simulink model "GLOSA_cs"  (Simulink 7.6)
settings: Solver=ode5  SolverMode=SingleTasking  StartTime=0.0  StopTime=40  FixedStep=1/100  RelTol=1e-3
PreLoadFcn:
  | check_NUTS_defined;
  | 
  | assignin('base',['model_closed_' bdroot],false);
InitFcn:
  | PreScanExperimentInitFcn(bdroot)
StopFcn:
  | PreScanExperimentStopFcn(bdroot)
undefined symbols (not defined in the file or in its folder): acceleration, actorID, actorType, DiscIntNeverNeededParam, GPS_alt, GPS_lat, GPS_long, intmax, lightBarInUse, messageID, publicVehicleType, rotZ, sireneInUse, trafficLightPriority, turnAdvice, velocity, x_ini_VRML, y_ini_VRML, yawRate, z_ini_VRML
libraries: simulink x337, simulink_extras x42, PreScanSimulinkLibrary x38, PreScanUsefulBlocks x14, simulink_need_slupdate x6
(part 38 of 38)

== stateflow chart Toyota_Previa_1/GLOSA/GLOSA ==
  state GLOSA [parallel]
    settings: executionOrder=1
    state dvVelocity
      (default) -> (junction 1368) : /* Velocity */
      (junction 1368) -> (junction 1370) [order 1] : [ (vForward == 1)]
      (junction 1370) -> (junction 1380) : {dvVelocity = CAM_Velocity[i];}
          | /* If car is at the front desired velocity if cars are set to its value */
      (junction 1368) -> (junction 1314) [order 2] : {dvVelocity = desiredVelocity;}
      (junction 1380) -> (junction 1314)
      (junction 1314) -> (junction 1381)
    state AngleandDistance
      state vDistance [parallel]
        settings: executionOrder=2
        (junction 1362) -> (junction 1361) : {Distance = 6371000*c;}
            | /*Calculate distance to the object */
        (default) -> (junction 1363) : {a = (sin((CAM_GPSLatitude[i]*d2r-selfGPSLatitude*d2r)/2 ))^2+ cos(selfGPSLatitude*d2r)*cos(CAM_GPSLatitude[i]*d2r)*(sin((CAM_GPSLongitude[i]*d2r-selfGPSLongitude*d2r)/2))^2;}
        (junction 1363) -> (junction 1362) : {c=2*atan2(sqrt(a), sqrt(1-a));}
      state vAngle [parallel]
        settings: executionOrder=1
        (default) -> (junction 1364) : {Angle = (atan2(CAM_GPSLatitude[i]-selfGPSLatitude, CAM_GPSLongitude[i] - selfGPSLongitude))/d2r;}
            | /* Calculate angle to the object */
    state diVelocity
      (default) -> (junction 1335) : /* Velocity */
      (junction 1316) -> (junction 1369)
      (junction 1335) -> (junction 1334) [order 1] : [ (iForward == 1)]
      (junction 1335) -> (junction 1316) [order 2] : {diVelocity = desiredVelocity;}
      (junction 1334) -> (junction 1367) : {diVelocity = (CAM_message[i] - 1000) /3.6;}
          | /* If sign is foreward, set desired infrastructure velocity to its limit */
      (junction 1367) -> (junction 1316)
    state vForward
      (junction 1320) -> (junction 1326) [order 1] : [((CAM_YawRate[i] > 2 || CAM_YawRate[i] <-2)  && (selfYawRate > 2 || selfYawRate < -2))]
      (junction 1326) -> (junction 1350) : {R1 = CAM_Velocity[i]/(CAM_YawRate[i]*d2r);
          | R2 = selfVelocity/(selfYawRate*d2r);
          | alfa = CAM_Heading[i]-selfHeading;
          | L = 2*d2r*180*R2*(alfa/360);}
      (junction 1387) -> (junction 1372)
      (junction 1371) -> (junction 1387) : {bend = 1;}
          | /* Cars are on the same bent if both have non-zero yaw rate and estimated agle at detected distance of the host car is similar to the angle of detected vehicle */
      (junction 1350) -> (junction 1372) [order 2] : {bend = 2;}
      (junction 1310) -> (junction 1313)
      (junction 1324) -> (junction 1311) [order 2]
      (junction 1372) -> (junction 1325)
      (junction 1325) -> (junction 1324)
      (junction 1313) -> (junction 1311) [order 2]
      (default) -> (junction 1320)
      (junction 1317) -> (junction 1318) [order 1] : [abs((Angle-selfHeading)%%360)<45 || abs((Angle-selfHeading)%%360)>315 || bend == 1]
      (junction 1366) -> (junction 1358) : {vForward = 0;}
      (junction 1318) -> (junction 1319) : {vForward = 1;}
          | /* Car is at the front if it will be reached in 2s and angles of cars are similar or they are on the bend */
      (junction 1324) -> (junction 1310) [order 1] : [(abs(CAM_Heading[i]-selfHeading)<45 || abs(CAM_Heading[i]-selfHeading)>315) || bend == 1 ]
      (junction 1317) -> (junction 1366) [order 2]
      (junction 1313) -> (junction 1315) [order 1] : [Distance < 100 && (selfVelocity - CAM_Velocity[i]) > 0 &&((Distance / (selfVelocity - CAM_Velocity[i])) < 2) && (abs(selfGPSAltitude - CAM_GPSAltitude[i]) < 10)]
      (junction 1320) -> (junction 1325) [order 2] : {bend = 0;}
      (junction 1315) -> (junction 1317)
      (junction 1311) -> (junction 1366)
      (junction 1350) -> (junction 1371) [order 1] : [((abs(L/selfVelocity*selfYawRate + selfHeading - CAM_Heading[i]) < 45) || (abs(L/selfVelocity*selfYawRate + selfHeading - CAM_Heading[i]) > 315)) ]
    state Velocity
      (junction 1340) -> (junction 1338) [order 2]
      (junction 1340) -> (junction 1339) [order 1] : [(dvVelocity<diVelocity) && (dvVelocity < desiredVelocity) && (vForward == 1)]
      (junction 1342) -> (junction 1340)
      (junction 1341) -> (junction 1342)
      (junction 1356) -> (junction 1341) : {Velocity = diVelocity;}
          | /* When Velocity from the sign is smaller then actual velocity of the car, Desired host velocity is set to the sign limit */
      (junction 1357) -> (junction 1342) [order 2] : {Velocity = desiredVelocity;}
      (junction 1357) -> (junction 1356) [order 1] : [(diVelocity<desiredVelocity)]
      (default) -> (junction 1357) : /* Velocity */
      (junction 1339) -> (junction 1337) : {Velocity = dvVelocity;}
          | /* When detected car hes smaller velocity then host car and sign limits, desired velocity of host car is set to velocity of detected car */
      (junction 1338) -> (junction 1336) : {Velocity = min(dvVelocity, diVelocity, dlVelocity, desiredVelocity);}
      (junction 1337) -> (junction 1338)
    state lForward
      note: IF condition1 THEN action1
          | ELSE action2
          | ENDIF
      (junction 1377) -> (junction 1322) : {lForward = 0;}
      (junction 1393) -> (junction 1323) : {lForward = 1;}
          | /* Light recognized as foreward one, when it is less then 500m away and Angle to it less then 45 deg.*/
      (junction 1375) -> (junction 1393) [order 1] : [abs((Angle-selfHeading) %% 360)<45 || abs((Angle-selfHeading)%% 360)>315]
      (junction 1375) -> (junction 1377) [order 2]
      (junction 1390) -> (junction 1373) [order 2]
      (junction 1374) -> (junction 1373) [order 2]
      (junction 1379) -> (junction 1375)
      (junction 1389) -> (junction 1374)
      (junction 1374) -> (junction 1379) [order 1] : [Distance < 500 && (abs(selfGPSAltitude - CAM_GPSAltitude[i]) < 10)]
      (junction 1373) -> (junction 1377)
      (junction 1390) -> (junction 1389) [order 1] : [abs(CAM_Heading[i]-selfHeading)<45 || abs(CAM_Heading[i]-selfHeading)>315]
      (default) -> (junction 1390)
    state iForward
      note: IF condition1 THEN action1
          | ELSE action2
          | ENDIF
      (junction 1312) -> (junction 1359) [order 1] : [Distance < 20 && (abs(selfGPSAltitude - CAM_GPSAltitude[i]) < 10)]
      (junction 1321) -> (junction 1347) [order 1] : [abs((Angle-selfHeading)%%360)<45 || abs((Angle-selfHeading)%%360)>315]
      (junction 1312) -> (junction 1346) [order 2]
      (junction 1347) -> (junction 1348) : {iForward = 1;}
          | /* Sign recognized as foreward one, when it is less then 20m away and Angle to it less then 45 deg.*/
      (junction 1346) -> (junction 1360)
      (junction 1359) -> (junction 1321)
      (junction 1360) -> (junction 1349) : {iForward = 0;}
      (junction 1321) -> (junction 1360) [order 2]
      (junction 1365) -> (junction 1355) [order 1] : [abs(CAM_Heading[i]-selfHeading)<45 || abs(CAM_Heading[i]-selfHeading)>315]
      (default) -> (junction 1365)
      (junction 1355) -> (junction 1312)
      (junction 1365) -> (junction 1346) [order 2]
    state dlVelocity
      (junction 1353) -> (junction 1351) : {dlVelocity = max((Distance-7)/(CAM_warning[i]+20), 0);}
          | /*dlVelocity = Distance/CAM_warning[i];}*/
      (junction 1384) -> (junction 1354) [order 2]
      (junction 1388) -> (junction 1391)
      (junction 1392) -> (junction 1388)
      (junction 1382) -> (junction 1388) [order 2] : {dlVelocity=desiredVelocity;}
      (junction 1378) -> (junction 1376)
      (junction 1386) -> (junction 1384) [order 2]
      (junction 1351) -> (junction 1392)
      (junction 1354) -> (junction 1353) [order 2]
      (junction 1354) -> (junction 1352) [order 1] : [TTL<CAM_warning[i]]
      (junction 1386) -> (junction 1385) [order 1] : [CAM_trafficLightPriority[i] == 3]
      (junction 1352) -> (junction 1376) : {dlVelocity= desiredVelocity;}
      (junction 1328) -> (junction 1378)
      (junction 1376) -> (junction 1351)
      (junction 1385) -> (junction 1328) : {dlVelocity=desiredVelocity;}
      (junction 1327) -> (junction 1378) : {dlVelocity = max((Distance-2)/(CAM_warning[i]+0.5), 0);}
      (junction 1384) -> (junction 1327) [order 1] : [CAM_trafficLightPriority[i] == 1]
      (default) -> (junction 1382) : /* Velocity */
      (junction 1382) -> (junction 1383) [order 1] : [ lForward == 1]
      (junction 1383) -> (junction 1386) : {TTL = Distance/selfVelocity;
          | Light = CAM_trafficLightPriority[i];
          | Time = CAM_warning[i];}
    lForward -> dlVelocity
    (junction 1330) -> AngleandDistance
    (default) -> (junction 1329) : {i =0;
        | vForward = 0;
        | iForward = 0;}
    (junction 1329) -> (junction 1330) [order 1] : [(vForward ==0 || iForward == 0) && i < CAM_Size]
        |  /*till sign and vehicle aren't detected*/
    (junction 1331) -> iForward [order 2] : [iForward == 0 && (CAM_vehicleType[i] == 14 || CAM_vehicleType[i] == 15) && CAM_message[i] > 1000]
        | /* If no traffic sign are detected and Velocity of the sign isn't 0 */
    (junction 1333) -> (junction 1329) : {i = 0;
        | vForward = 0;
        | iForward = 0;
        | lForward = 0;}
    (junction 1343) -> (junction 1329) : {i++;}
    (junction 1331) -> (junction 1332) [order 3]
    (junction 1329) -> Velocity [order 2]
    (junction 1332) -> vForward [order 1] : [vForward == 0 && CAM_vehicleType[i] != 0]
        | /* If there was no nearer car at the front and Vehicle Type ID isn't 0 */
    (junction 1332) -> (junction 1343) [order 2]
    iForward -> diVelocity
    Velocity -> (junction 1333)
    vForward -> dvVelocity
    dvVelocity -> (junction 1345)
    diVelocity -> (junction 1345)
    (junction 1344) -> (junction 1343)
    (junction 1345) -> (junction 1344)
    AngleandDistance -> (junction 1331)
    (junction 1331) -> lForward [order 1] : [lForward == 0 && CAM_trafficLightPriority[i] !=0]
    dlVelocity -> (junction 1345)
  note: GLOSA Algorithms Based on CAM signal changed to continous
  data: R1 local double; R2 local double; a local double; alfa local double; bend local double; c local double; diVelocity local double =100; dvVelocity local double =100; i local double; iForward local double; L local double; vForward local double; dlVelocity local double =100; selfHeading input double; selfGPSLongitude input double; selfGPSLatitude input double; selfGPSAltitude input double; selfYawRate input double; selfVelocity input double; CAM_warning input double; CAM_message input double; CAM_vehicleType input double; Velocity output double; CAM_Velocity input double; CAM_YawRate input double; CAM_trafficLightPriority input double; CAM_GPSLatitude input double; CAM_GPSLongitude input double; CAM_GPSAltitude input double; CAM_Heading input double; CAM_Size input double; d2r constant double =pi()/180; desiredVelocity input double; TTL local double; lForward output double; Angle local double; Distance local double; Time output double; Light output double

== matlab function Toyota_Previa_1/GLOSA/vehicle CAM generator/For Iterator Subsystem/Continuous signal ==
  data: CAM input double; CAM_o output double; CAM_old input double; i input double; NoMM input double; tmp_in input double; tmp_out output double
function [CAM_o, tmp_out] = con(CAM, CAM_old, i, NoMM, tmp_in)
%linearization and number of missed frames control
CAM_o = zeros(1, 16);
tmp_out = tmp_in(i);
if (CAM(i, 3) ~= 0)
    if (mod(tmp_in(i), NoMM+1) == 0)
        CAM_o = CAM (i, :);
    end
    tmp_out = tmp_in(i)+1;
else
    CAM_o = CAM_old(i, :);
end

== matlab function Toyota_Previa_1/GLOSA/vehicle CAM generator/Seperate signals ==
  data: CAM_warning output double; CAM input double; CAM_messageID output double; CAM_vehicleID output double; CAM_vehicleType output double; CAM_publicVehicleType output double; CAM_lightBarInUse output double; CAM_sireneInUse output double; CAM_velocity output double; CAM_acceleration output double; CAM_yawRate output double; CAM_turnAdvice output double; CAM_trafficLightPriority output double; CAM_GPSlat output double; CAM_GPSlong output double; CAM_GPSalt output double; CAM_rotZ output double
function [CAM_warning, CAM_messageID, CAM_vehicleID, CAM_vehicleType, CAM_publicVehicleType, CAM_lightBarInUse, CAM_sireneInUse, CAM_velocity, CAM_acceleration, CAM_yawRate, CAM_turnAdvice, CAM_trafficLightPriority, CAM_GPSlat, CAM_GPSlong, CAM_GPSalt, CAM_rotZ] = fcn(CAM)
%Seperate signals

CAM_warning = CAM(:, 1);
CAM_messageID = CAM(:, 2); 
CAM_vehicleID = CAM(:, 3);
CAM_vehicleType = CAM(:, 4);
CAM_publicVehicleType = CAM(:, 5);
CAM_lightBarInUse = CAM(:, 6);
CAM_sireneInUse = CAM(:, 7);
CAM_velocity = CAM(:, 8);
CAM_acceleration = CAM(:, 9);
CAM_yawRate = CAM(:, 10);
CAM_turnAdvice = CAM(:, 11);
CAM_trafficLightPriority = CAM(:, 12);
CAM_GPSlat = CAM(:, 13);
CAM_GPSlong = CAM(:, 14);
CAM_GPSalt = CAM(:, 15);
CAM_rotZ = CAM(:, 16);

== matlab function Toyota_Previa_1/GLOSA/vehicle CAM generator/Signal Matrix create ==
  data: CAM_warning input double; CAM output double; CAM_messageID input double; CAM_vehicleID input double; CAM_vehicleType input double; CAM_publicVehicleType input double; CAM_lightBarInUse input double; CAM_sireneInUse input double; CAM_velocity input double; CAM_acceleration input double; CAM_yawRate input double; CAM_turnAdvice input double; CAM_trafficLightPriority input double; CAM_GPSlat input double; CAM_GPSlong input double; CAM_GPSalt input double; CAM_rotZ input double
function CAM = fcn(CAM_warning, CAM_messageID, CAM_vehicleID, CAM_vehicleType, CAM_publicVehicleType, CAM_lightBarInUse, CAM_sireneInUse, CAM_velocity, CAM_acceleration, CAM_yawRate, CAM_turnAdvice, CAM_trafficLightPriority, CAM_GPSlat, CAM_GPSlong, CAM_GPSalt, CAM_rotZ)
%Signal Matrix creation

CAM = [CAM_warning, CAM_messageID, CAM_vehicleID, CAM_vehicleType, CAM_publicVehicleType, CAM_lightBarInUse, CAM_sireneInUse, CAM_velocity, CAM_acceleration, CAM_yawRate, CAM_turnAdvice, CAM_trafficLightPriority, CAM_GPSlat, CAM_GPSlong, CAM_GPSalt, CAM_rotZ];

== matlab function Ford_Fiesta_1/GLOSA/Embedded MATLAB Function ==
  data: u input double; y output double
function y = fcn(u)
%#eml

y = mod(u, 360);

== stateflow chart Ford_Fiesta_1/GLOSA/GLOSA ==
  state GLOSA [parallel]
    settings: executionOrder=1
    state dvVelocity
      (default) -> (junction 1683) : /* Velocity */
      (junction 1683) -> (junction 1685) [order 1] : [ (vForward == 1)]
      (junction 1685) -> (junction 1695) : {dvVelocity = CAM_Velocity[i];}
          | /* If car is at the front desired velocity if cars are set to its value */
      (junction 1683) -> (junction 1629) [order 2] : {dvVelocity = desiredVelocity;}
      (junction 1695) -> (junction 1629)
      (junction 1629) -> (junction 1696)
    state AngleandDistance
      state vDistance [parallel]
        settings: executionOrder=2
        (junction 1677) -> (junction 1676) : {Distance = 6371000*c;}
            | /*Calculate distance to the object */
        (default) -> (junction 1678) : {a = (sin((CAM_GPSLatitude[i]*d2r-selfGPSLatitude*d2r)/2 ))^2+ cos(selfGPSLatitude*d2r)*cos(CAM_GPSLatitude[i]*d2r)*(sin((CAM_GPSLongitude[i]*d2r-selfGPSLongitude*d2r)/2))^2;}
        (junction 1678) -> (junction 1677) : {c=2*atan2(sqrt(a), sqrt(1-a));}
      state vAngle [parallel]
        settings: executionOrder=1
        (default) -> (junction 1679) : {Angle = (atan2(CAM_GPSLatitude[i]-selfGPSLatitude, CAM_GPSLongitude[i] - selfGPSLongitude))/d2r;}
            | /* Calculate angle to the object */
    state diVelocity
      (default) -> (junction 1650) : /* Velocity */
      (junction 1631) -> (junction 1684)
      (junction 1650) -> (junction 1649) [order 1] : [ (iForward == 1)]
      (junction 1650) -> (junction 1631) [order 2] : {diVelocity = desiredVelocity;}
      (junction 1649) -> (junction 1682) : {diVelocity = (CAM_message[i] - 1000) /3.6;}
          | /* If sign is foreward, set desired infrastructure velocity to its limit */
      (junction 1682) -> (junction 1631)
    state vForward
      (junction 1635) -> (junction 1641) [order 1] : [((CAM_YawRate[i] > 2 || CAM_YawRate[i] <-2)  && (selfYawRate > 2 || selfYawRate < -2))]
      (junction 1641) -> (junction 1665) : {R1 = CAM_Velocity[i]/(CAM_YawRate[i]*d2r);
          | R2 = selfVelocity/(selfYawRate*d2r);
          | alfa = CAM_Heading[i]-selfHeading;
          | L = 2*d2r*180*R2*(alfa/360);}
      (junction 1702) -> (junction 1687)
      (junction 1686) -> (junction 1702) : {bend = 1;}
          | /* Cars are on the same bent if both have non-zero yaw rate and estimated agle at detected distance of the host car is similar to the angle of detected vehicle */
      (junction 1665) -> (junction 1687) [order 2] : {bend = 2;}
      (junction 1625) -> (junction 1628)
      (junction 1639) -> (junction 1626) [order 2]
      (junction 1687) -> (junction 1640)
      (junction 1640) -> (junction 1639)
      (junction 1628) -> (junction 1626) [order 2]
      (default) -> (junction 1635)
      (junction 1632) -> (junction 1633) [order 1] : [abs((Angle-selfHeading)%%360)<45 || abs((Angle-selfHeading)%%360)>315 || bend == 1]
      (junction 1681) -> (junction 1673) : {vForward = 0;}
      (junction 1633) -> (junction 1634) : {vForward = 1;}
          | /* Car is at the front if it will be reached in 2s and angles of cars are similar or they are on the bend */
      (junction 1639) -> (junction 1625) [order 1] : [(abs(CAM_Heading[i]-selfHeading)<45 || abs(CAM_Heading[i]-selfHeading)>315) || bend == 1 ]
      (junction 1632) -> (junction 1681) [order 2]
      (junction 1628) -> (junction 1630) [order 1] : [Distance < 100 && (selfVelocity - CAM_Velocity[i]) > 0 &&((Distance / (selfVelocity - CAM_Velocity[i])) < 2) && (abs(selfGPSAltitude - CAM_GPSAltitude[i]) < 10)]
      (junction 1635) -> (junction 1640) [order 2] : {bend = 0;}
      (junction 1630) -> (junction 1632)
      (junction 1626) -> (junction 1681)
      (junction 1665) -> (junction 1686) [order 1] : [((abs(L/selfVelocity*selfYawRate + selfHeading - CAM_Heading[i]) < 45) || (abs(L/selfVelocity*selfYawRate + selfHeading - CAM_Heading[i]) > 315)) ]
    state Velocity
      (junction 1655) -> (junction 1653) [order 2]
      (junction 1655) -> (junction 1654) [order 1] : [(dvVelocity<diVelocity) && (dvVelocity < desiredVelocity) && (vForward == 1)]
      (junction 1657) -> (junction 1655)
      (junction 1656) -> (junction 1657)
      (junction 1671) -> (junction 1656) : {Velocity = diVelocity;}
          | /* When Velocity from the sign is smaller then actual velocity of the car, Desired host velocity is set to the sign limit */
      (junction 1672) -> (junction 1657) [order 2] : {Velocity = desiredVelocity;}
      (junction 1672) -> (junction 1671) [order 1] : [(diVelocity<desiredVelocity)]
      (default) -> (junction 1672) : /* Velocity */
      (junction 1654) -> (junction 1652) : {Velocity = dvVelocity;}
          | /* When detected car hes smaller velocity then host car and sign limits, desired velocity of host car is set to velocity of detected car */
      (junction 1653) -> (junction 1651) : {Velocity = min(dvVelocity, diVelocity, dlVelocity, desiredVelocity);}
      (junction 1652) -> (junction 1653)
    state lForward
      note: IF condition1 THEN action1
          | ELSE action2
          | ENDIF
      (junction 1692) -> (junction 1637) : {lForward = 0;}
      (junction 1708) -> (junction 1638) : {lForward = 1;}
          | /* Light recognized as foreward one, when it is less then 500m away and Angle to it less then 45 deg.*/
      (junction 1690) -> (junction 1708) [order 1] : [abs((Angle-selfHeading) %% 360)<45 || abs((Angle-selfHeading)%% 360)>315]
      (junction 1690) -> (junction 1692) [order 2]
      (junction 1705) -> (junction 1688) [order 2]
      (junction 1689) -> (junction 1688) [order 2]
      (junction 1694) -> (junction 1690)
      (junction 1704) -> (junction 1689)
      (junction 1689) -> (junction 1694) [order 1] : [Distance < 500 && (abs(selfGPSAltitude - CAM_GPSAltitude[i]) < 10)]
      (junction 1688) -> (junction 1692)
      (junction 1705) -> (junction 1704) [order 1] : [abs(CAM_Heading[i]-selfHeading)<45 || abs(CAM_Heading[i]-selfHeading)>315]
      (default) -> (junction 1705)
    state iForward
      note: IF condition1 THEN action1
          | ELSE action2
          | ENDIF
      (junction 1627) -> (junction 1674) [order 1] : [Distance < 20 && (abs(selfGPSAltitude - CAM_GPSAltitude[i]) < 10)]
      (junction 1636) -> (junction 1662) [order 1] : [abs((Angle-selfHeading)%%360)<45 || abs((Angle-selfHeading)%%360)>315]
      (junction 1627) -> (junction 1661) [order 2]
      (junction 1662) -> (junction 1663) : {iForward = 1;}
          | /* Sign recognized as foreward one, when it is less then 20m away and Angle to it less then 45 deg.*/
      (junction 1661) -> (junction 1675)
      (junction 1674) -> (junction 1636)
      (junction 1675) -> (junction 1664) : {iForward = 0;}
      (junction 1636) -> (junction 1675) [order 2]
      (junction 1680) -> (junction 1670) [order 1] : [abs(CAM_Heading[i]-selfHeading)<45 || abs(CAM_Heading[i]-selfHeading)>315]
      (default) -> (junction 1680)
      (junction 1670) -> (junction 1627)
      (junction 1680) -> (junction 1661) [order 2]
    state dlVelocity
      (junction 1668) -> (junction 1666) : {dlVelocity = max((Distance-7)/(CAM_warning[i]+20), 0);}
          | /*dlVelocity = Distance/CAM_warning[i];}*/
      (junction 1699) -> (junction 1669) [order 2]
      (junction 1703) -> (junction 1706)
      (junction 1707) -> (junction 1703)
      (junction 1697) -> (junction 1703) [order 2] : {dlVelocity=desiredVelocity;}
      (junction 1693) -> (junction 1691)
      (junction 1701) -> (junction 1699) [order 2]
      (junction 1666) -> (junction 1707)
      (junction 1669) -> (junction 1668) [order 2]
      (junction 1669) -> (junction 1667) [order 1] : [TTL<CAM_warning[i]]
      (junction 1701) -> (junction 1700) [order 1] : [CAM_trafficLightPriority[i] == 3]
      (junction 1667) -> (junction 1691) : {dlVelocity= desiredVelocity;}
      (junction 1643) -> (junction 1693)
      (junction 1691) -> (junction 1666)
      (junction 1700) -> (junction 1643) : {dlVelocity=desiredVelocity;}
      (junction 1642) -> (junction 1693) : {dlVelocity = max((Distance-2)/(CAM_warning[i]+0.5), 0);}
      (junction 1699) -> (junction 1642) [order 1] : [CAM_trafficLightPriority[i] == 1]
      (default) -> (junction 1697) : /* Velocity */
      (junction 1697) -> (junction 1698) [order 1] : [ lForward == 1]
      (junction 1698) -> (junction 1701) : {TTL = Distance/selfVelocity;
          | Light = CAM_trafficLightPriority[i];
          | Time = CAM_warning[i];}
    lForward -> dlVelocity
    (junction 1645) -> AngleandDistance
    (default) -> (junction 1644) : {i =0;
        | vForward = 0;
        | iForward = 0;}
    (junction 1644) -> (junction 1645) [order 1] : [(vForward ==0 || iForward == 0) && i < CAM_Size]
        |  /*till sign and vehicle aren't detected*/
    (junction 1646) -> iForward [order 2] : [iForward == 0 && (CAM_vehicleType[i] == 14 || CAM_vehicleType[i] == 15) && CAM_message[i] > 1000]
        | /* If no traffic sign are detected and Velocity of the sign isn't 0 */
    (junction 1648) -> (junction 1644) : {i = 0;
        | vForward = 0;
        | iForward = 0;
        | lForward = 0;}
    (junction 1658) -> (junction 1644) : {i++;}
    (junction 1646) -> (junction 1647) [order 3]
    (junction 1644) -> Velocity [order 2]
    (junction 1647) -> vForward [order 1] : [vForward == 0 && CAM_vehicleType[i] != 0]
        | /* If there was no nearer car at the front and Vehicle Type ID isn't 0 */
    (junction 1647) -> (junction 1658) [order 2]
    iForward -> diVelocity
    Velocity -> (junction 1648)
    vForward -> dvVelocity
    dvVelocity -> (junction 1660)
    diVelocity -> (junction 1660)
    (junction 1659) -> (junction 1658)
    (junction 1660) -> (junction 1659)
    AngleandDistance -> (junction 1646)
    (junction 1646) -> lForward [order 1] : [lForward == 0 && CAM_trafficLightPriority[i] !=0]
    dlVelocity -> (junction 1660)
  note: GLOSA Algorithms Based on CAM signal changed to continous
  data: R1 local double; R2 local double; a local double; alfa local double; bend local double; c local double; diVelocity local double =100; dvVelocity local double =100; i local double; iForward local double; L local double; vForward local double; dlVelocity local double =100; selfHeading input double; selfGPSLongitude input double; selfGPSLatitude input double; selfGPSAltitude input double; selfYawRate input double; selfVelocity input double; CAM_warning input double; CAM_message input double; CAM_vehicleType input double; Velocity output double; CAM_Velocity input double; CAM_YawRate input double; CAM_trafficLightPriority input double; CAM_GPSLatitude input double; CAM_GPSLongitude input double; CAM_GPSAltitude input double; CAM_Heading input double; CAM_Size input double; d2r constant double =pi()/180; desiredVelocity input double; TTL local double; lForward output double; Angle local double; Distance local double; Time output double; Light output double

== matlab function Ford_Fiesta_1/GLOSA/vehicle CAM generator/For Iterator Subsystem/Continuous signal ==
  data: CAM input double; CAM_o output double; CAM_old input double; i input double; NoMM input double; tmp_in input double; tmp_out output double
function [CAM_o, tmp_out] = con(CAM, CAM_old, i, NoMM, tmp_in)
%linearization and number of missed frames control
CAM_o = zeros(1, 16);
tmp_out = tmp_in(i);
if (CAM(i, 3) ~= 0)
    if (mod(tmp_in(i), NoMM+1) == 0)
        CAM_o = CAM (i, :);
    end
    tmp_out = tmp_in(i)+1;
else
    CAM_o = CAM_old(i, :);
end

== matlab function Ford_Fiesta_1/GLOSA/vehicle CAM generator/Seperate signals ==
  data: CAM_warning output double; CAM input double; CAM_messageID output double; CAM_vehicleID output double; CAM_vehicleType output double; CAM_publicVehicleType output double; CAM_lightBarInUse output double; CAM_sireneInUse output double; CAM_velocity output double; CAM_acceleration output double; CAM_yawRate output double; CAM_turnAdvice output double; CAM_trafficLightPriority output double; CAM_GPSlat output double; CAM_GPSlong output double; CAM_GPSalt output double; CAM_rotZ output double
function [CAM_warning, CAM_messageID, CAM_vehicleID, CAM_vehicleType, CAM_publicVehicleType, CAM_lightBarInUse, CAM_sireneInUse, CAM_velocity, CAM_acceleration, CAM_yawRate, CAM_turnAdvice, CAM_trafficLightPriority, CAM_GPSlat, CAM_GPSlong, CAM_GPSalt, CAM_rotZ] = fcn(CAM)
%Seperate signals

CAM_warning = CAM(:, 1);
CAM_messageID = CAM(:, 2); 
CAM_vehicleID = CAM(:, 3);
CAM_vehicleType = CAM(:, 4);
CAM_publicVehicleType = CAM(:, 5);
CAM_lightBarInUse = CAM(:, 6);
CAM_sireneInUse = CAM(:, 7);
CAM_velocity = CAM(:, 8);
CAM_acceleration = CAM(:, 9);
CAM_yawRate = CAM(:, 10);
CAM_turnAdvice = CAM(:, 11);
CAM_trafficLightPriority = CAM(:, 12);
CAM_GPSlat = CAM(:, 13);
CAM_GPSlong = CAM(:, 14);
CAM_GPSalt = CAM(:, 15);
CAM_rotZ = CAM(:, 16);

== matlab function Mazda_RX8_1/GLOSA/vehicle CAM generator/Signal Matrix create ==
  data: CAM_warning input double; CAM output double; CAM_messageID input double; CAM_vehicleID input double; CAM_vehicleType input double; CAM_publicVehicleType input double; CAM_lightBarInUse input double; CAM_sireneInUse input double; CAM_velocity input double; CAM_acceleration input double; CAM_yawRate input double; CAM_turnAdvice input double; CAM_trafficLightPriority input double; CAM_GPSlat input double; CAM_GPSlong input double; CAM_GPSalt input double; CAM_rotZ input double
function CAM = fcn(CAM_warning, CAM_messageID, CAM_vehicleID, CAM_vehicleType, CAM_publicVehicleType, CAM_lightBarInUse, CAM_sireneInUse, CAM_velocity, CAM_acceleration, CAM_yawRate, CAM_turnAdvice, CAM_trafficLightPriority, CAM_GPSlat, CAM_GPSlong, CAM_GPSalt, CAM_rotZ)
%Signal Matrix creation

CAM = [CAM_warning, CAM_messageID, CAM_vehicleID, CAM_vehicleType, CAM_publicVehicleType, CAM_lightBarInUse, CAM_sireneInUse, CAM_velocity, CAM_acceleration, CAM_yawRate, CAM_turnAdvice, CAM_trafficLightPriority, CAM_GPSlat, CAM_GPSlong, CAM_GPSalt, CAM_rotZ];

== matlab function Ford_Fiesta_1/GLOSA/vehicle CAM generator/Signal Matrix create ==
  data: CAM_warning input double; CAM output double; CAM_messageID input double; CAM_vehicleID input double; CAM_vehicleType input double; CAM_publicVehicleType input double; CAM_lightBarInUse input double; CAM_sireneInUse input double; CAM_velocity input double; CAM_acceleration input double; CAM_yawRate input double; CAM_turnAdvice input double; CAM_trafficLightPriority input double; CAM_GPSlat input double; CAM_GPSlong input double; CAM_GPSalt input double; CAM_rotZ input double
function CAM = fcn(CAM_warning, CAM_messageID, CAM_vehicleID, CAM_vehicleType, CAM_publicVehicleType, CAM_lightBarInUse, CAM_sireneInUse, CAM_velocity, CAM_acceleration, CAM_yawRate, CAM_turnAdvice, CAM_trafficLightPriority, CAM_GPSlat, CAM_GPSlong, CAM_GPSalt, CAM_rotZ)
%Signal Matrix creation

CAM = [CAM_warning, CAM_messageID, CAM_vehicleID, CAM_vehicleType, CAM_publicVehicleType, CAM_lightBarInUse, CAM_sireneInUse, CAM_velocity, CAM_acceleration, CAM_yawRate, CAM_turnAdvice, CAM_trafficLightPriority, CAM_GPSlat, CAM_GPSlong, CAM_GPSalt, CAM_rotZ];

== matlab function Embedded MATLAB Function2 ==
  data: red_time input double; lightID output double; lightID2 output double; yellow_time input double; green_time input double; both_red input double; time_delay input double; time input double; last_time input double; red output double; red2 output double; yellow output double; yellow2 output double; green output double; green2 output double; light_time output double; light_time2 output double; last_time_out output double
function [lightID, lightID2, red, red2, yellow, yellow2, green, green2, light_time, light_time2, last_time_out] = fcn(red_time, yellow_time, green_time, both_red, time_delay, time, last_time)
%#eml
total_time = red_time + yellow_time + green_time;
green_time2 = red_time - 2*both_red - 3*yellow_time;
red_time2 = green_time + 3*yellow_time + 2*both_red;
time_delay = mod(time_delay, total_time);
time2 = (time - last_time + time_delay);
if ((time2) <= green_time)
    green = 1;
    green2 = 0;
    yellow = 0;
    yellow2 = 0;
    red = 0;
    red2 = 1;
    lightID = 3;
    lightID2 = 1;
    last_time_out = last_time;
    light_time = green_time - time2;
    light_time2 = red_time2 - time2 - both_red - yellow_time;
elseif((time2) <= (green_time+yellow_time))
    green = 0;
    green2 = 0;
    yellow = 1;
    yellow2 = 0;
    red = 0;
    red2 = 1;
    lightID = 2;
    lightID2 = 1;
    last_time_out = last_time;
    light_time = yellow_time - (time2 - green_time);
    light_time2 = red_time2 - time2 - both_red - yellow_time;
elseif((time2) <= (green_time+yellow_time+both_red))
    green = 0;
    green2 = 0;
    yellow = 0;
    yellow2 = 0;
    red = 1;
    red2 = 1;
    lightID = 1;
    lightID2 = 1;
    last_time_out = last_time;
    light_time = red_time - (time2 - green_time - yellow_time);
    light_time2 = red_time2 - time2 - both_red - yellow_time;
elseif((time2) <= (green_time + 2*yellow_time + both_red))
    green = 0;
    green2 = 0;
    yellow = 0;
    yellow2 = 1;
    red = 1;
    red2 = 1;
    lightID = 1;
    lightID2 = 1;
    last_time_out = last_time;
    light_time = red_time - (time2 - green_time - yellow_time);
    light_time2 = red_time2 - time2 - both_red - yellow_time;
elseif((time2) <= (green_time+2*yellow_time + both_red + green_time2))
    green = 0;
    green2 = 1;
    yellow = 0;
    yellow2 =0;
    red = 1;
    red2 = 0;
    lightID = 1;
    lightID2 = 3;
    last_time_out = last_time;
    light_time = red_time - (time2 - green_time - yellow_time);
    light_time2 = green_time2 - (time2 - red_time2 + both_red + yellow_time );
elseif((time2) <= (green_time+3*yellow_time + both_red + green_time2))
    green = 0;
    green2 = 0;
    yellow = 0;
    yellow2 =1;
    red = 1;
    red2 = 0;
    lightID = 1;
    lightID2 = 2;
    last_time_out = last_time;
    light_time = red_time - (time2 - green_time - yellow_time);
    light_time2 = yellow_time - (time2 - red_time2 - green_time2 + both_red + yellow_time);
elseif((time2) <= (total_time - yellow_time))
    green = 0;
    green2 = 0;
    yellow = 0;
    yellow2 =0;
    red = 1;
    red2 = 1;
    lightID = 1;
    lightID2 = 1;
    last_time_out = last_time;
    light_time = red_time - (time2 - green_time - yellow_time);
    light_time2 = yellow_time - (time2 - red_time2 - green_time2 + both_red + yellow_time);
elseif((time2) < (total_time))
    green = 0;
    green2 = 0;
    yellow = 1;
    yellow2 =0;
    red = 1;
    red2 = 1;
    lightID = 1;
    lightID2 = 1;
    last_time_out = last_time;
    light_time = red_time - (time2 - green_time - yellow_time);
    light_time2 = total_time + red_time2 - both_red - yellow_time - time2;
else
    green = 0;
    green2 = 0;
    yellow = 1;
    yellow2 =0;
    red = 1;
    red2 = 1;
    lightID = 1;
    lightID2 = 1;
    last_time_out = time + time_delay;
    light_time = green_time - (time2 - green_time - yellow_time - red_time);
    light_time2 = total_time + red_time2 - both_red - yellow_time - time2;
end
